simulink model "Simulatore_COMAU_UBHAND_IV_manipolazione_interna"  (Simulink 7.6)
settings: Solver=ode45  StartTime=0.0  StopTime=inf  RelTol=1e-3
values from neighbouring files (symbols this model uses):
  a_f = zeros(4,5)    [KinematicData.m]
  alpha_f = zeros(4,5)    [KinematicData.m]
  d_f = zeros(4,5)    [KinematicData.m]
  dc4_1 = 660    [KinematicData.m]
  T6HAND = [1 0 0 0;0 1 0 0; 0 0 1 357.48+95; 0 0 0 1]    [KinematicData.m]
  teta_d = atan(647.07/130)    [KinematicData.m]
  teta_f = zeros(4,5)    [KinematicData.m]
undefined symbols (not defined in the file or in its folder): a_c, a_w, alpha_c, alpha_w, d_c, d_w, Dt_fingers, teta_c
libraries: simulink x281, dspmtrx3 x171, aerolibutil x163, aerolibconvert2 x64, simulink_extras x46, vrlib x26, aerolibtransform2 x7, dspnetwork x1
(part 11 of 15)

== system Simulatore_COMAU_UBHAND_IV_manipolazione_interna/Work Space Control/Inverse_kinematic/Inverse_kinematic_comau/Subsystem/q5 appartiene a [-180 0]/q6 ==
  R_36 : Inport
  Gain1 : Gain  Gain=-1
  P06(1) : dspmtrx3/Submatrix  RowSpan=One row  RowStartMode=Index  RowStartIndex=3  RowEndMode=Index  RowEndIndex=3  ColSpan=One column  ColStartMode=Index  ColStartIndex=2  ColEndMode=Offset from last  ColEndIndex=1
  P06(1)1 : dspmtrx3/Submatrix  RowSpan=One row  RowStartMode=Index  RowStartIndex=3  RowEndMode=Index  RowEndIndex=3  ColSpan=One column  ColStartMode=Index  ColStartIndex=1  ColEndMode=Offset from last  ColEndIndex=1
  Trigonometric Function : Trigonometry  Operator=atan2
  q_6 : Outport
  wires:
  Trigonometric Function/1 -> q_6/1
  P06(1)/1 -> Gain1/1
  P06(1)1/1 -> Trigonometric Function/2
  R_36/1 -> P06(1)1/1, P06(1)/1
  Gain1/1 -> Trigonometric Function/1

== system Simulatore_COMAU_UBHAND_IV_manipolazione_interna/Work Space Control/Inverse_kinematic/Inverse_kinematic_comau/Subsystem/q5 appartiene a [0 180]/q4 ==
  R_36 : Inport
  P06(1) : dspmtrx3/Submatrix  RowSpan=One row  RowStartMode=Index  RowStartIndex=2  RowEndMode=Index  RowEndIndex=3  ColSpan=One column  ColStartMode=Index  ColStartIndex=3  ColEndMode=Offset from last  ColEndIndex=1
  P06(1)1 : dspmtrx3/Submatrix  RowSpan=One row  RowStartMode=Index  RowStartIndex=1  RowEndMode=Index  RowEndIndex=3  ColSpan=One column  ColStartMode=Index  ColStartIndex=3  ColEndMode=Offset from last  ColEndIndex=1
  Trigonometric Function : Trigonometry  Operator=atan2
  q_4 : Outport
  wires:
  R_36/1 -> P06(1)/1, P06(1)1/1
  P06(1)/1 -> Trigonometric Function/1
  P06(1)1/1 -> Trigonometric Function/2
  Trigonometric Function/1 -> q_4/1

== system Simulatore_COMAU_UBHAND_IV_manipolazione_interna/Work Space Control/Inverse_kinematic/Inverse_kinematic_comau/Subsystem/q5 appartiene a [0 180]/q5 ==
  R_36 : Inport
  Add : Sum
  Math Function : Math  Operator=sqrt  SaturateOnIntegerOverflow=on
  Math Function1 : Math  Operator=square  SaturateOnIntegerOverflow=on
  Math Function2 : Math  Operator=square  SaturateOnIntegerOverflow=on
  P06(1) : dspmtrx3/Submatrix  RowSpan=One row  RowStartMode=Index  RowStartIndex=1  RowEndMode=Index  RowEndIndex=3  ColSpan=One column  ColStartMode=Index  ColStartIndex=3  ColEndMode=Offset from last  ColEndIndex=1
  P06(1)1 : dspmtrx3/Submatrix  RowSpan=One row  RowStartMode=Index  RowStartIndex=2  RowEndMode=Index  RowEndIndex=3  ColSpan=One column  ColStartMode=Index  ColStartIndex=3  ColEndMode=Offset from last  ColEndIndex=1
  P06(1)2 : dspmtrx3/Submatrix  RowSpan=One row  RowStartMode=Index  RowStartIndex=3  RowEndMode=Index  RowEndIndex=3  ColSpan=One column  ColStartMode=Index  ColStartIndex=3  ColEndMode=Offset from last  ColEndIndex=1
  Trigonometric Function : Trigonometry  Operator=atan2
  q_5 : Outport
  wires:
  P06(1)1/1 -> Math Function2/1
  P06(1)/1 -> Math Function1/1
  R_36/1 -> P06(1)/1, P06(1)1/1, P06(1)2/1
  Math Function1/1 -> Add/1
  Math Function2/1 -> Add/2
  Add/1 -> Math Function/1
  P06(1)2/1 -> Trigonometric Function/2
  Math Function/1 -> Trigonometric Function/1
  Trigonometric Function/1 -> q_5/1

== system Simulatore_COMAU_UBHAND_IV_manipolazione_interna/Work Space Control/Inverse_kinematic/Inverse_kinematic_comau/Subsystem/q5 appartiene a [0 180]/q6 ==
  R_36 : Inport
  Gain : Gain  Gain=-1
  P06(1) : dspmtrx3/Submatrix  RowSpan=One row  RowStartMode=Index  RowStartIndex=3  RowEndMode=Index  RowEndIndex=3  ColSpan=One column  ColStartMode=Index  ColStartIndex=2  ColEndMode=Offset from last  ColEndIndex=1
  P06(1)1 : dspmtrx3/Submatrix  RowSpan=One row  RowStartMode=Index  RowStartIndex=3  RowEndMode=Index  RowEndIndex=3  ColSpan=One column  ColStartMode=Index  ColStartIndex=1  ColEndMode=Offset from last  ColEndIndex=1
  Trigonometric Function : Trigonometry  Operator=atan2
  q_6 : Outport
  wires:
  R_36/1 -> P06(1)/1, P06(1)1/1
  P06(1)1/1 -> Gain/1
  Gain/1 -> Trigonometric Function/2
  P06(1)/1 -> Trigonometric Function/1
  Trigonometric Function/1 -> q_6/1

== system Simulatore_COMAU_UBHAND_IV_manipolazione_interna/Work Space Control/Inverse_kinematic/Inverse_kinematic_hand/Inverse_kinematic_little/Direct_kinematic_index/Subsystem ==
  q : Inport
  alpha : Inport  Port=2
  a : Inport  Port=3
  A_01 : aerolibutil/Create 3x3 Matrix
  Constant : Constant  Value=0
  Constant1 : Constant  Value=[0 0 0 1]  VectorParams1D=off
  Constant5 : Constant  Value=0
  Display : Display
  Gain : Gain  Gain=-1
  Gain1 : Gain  Gain=-1
  Matrix Concatenation : Concatenate  Mode=Multidimensional array  ConcatenateDimension=2
  Matrix Concatenation1 : Concatenate  Mode=Multidimensional array
  Product{,1..5} (6 alike) : Product  RndMeth=Zero
  Trigonometric Function{,1,6..8,11..12} (7 alike) : Trigonometry  Operator=cos
  Trigonometric Function{2..5,9..10,13} (7 alike) : Trigonometry
  Vector Concatenate : Concatenate  NumInputs=3  Mode=Multidimensional array
  Out1 : Outport
  wires:
  Matrix Concatenation1/1 -> Display/1, Out1/1
  Constant1/1 -> Matrix Concatenation1/2
  Matrix Concatenation/1 -> Matrix Concatenation1/1
  Vector Concatenate/1 -> Matrix Concatenation/2
  A_01/1 -> Matrix Concatenation/1
  Constant5/1 -> Vector Concatenate/3
  Product5/1 -> Vector Concatenate/2
  Product4/1 -> Vector Concatenate/1
  Trigonometric Function13/1 -> Product5/2
  Trigonometric Function12/1 -> Product4/2
  a/1 -> Product5/1, Product4/1
  Trigonometric Function11/1 -> A_01/9
  Trigonometric Function10/1 -> A_01/8
  Constant/1 -> A_01/7
  Gain1/1 -> A_01/6
  Product2/1 -> A_01/5
  Trigonometric Function5/1 -> A_01/4
  Product1/1 -> A_01/3
  Gain/1 -> A_01/2
  Trigonometric Function/1 -> A_01/1
  Product/1 -> Gain/1
  Product3/1 -> Gain1/1
  Trigonometric Function9/1 -> Product3/2
  Trigonometric Function8/1 -> Product3/1
  Trigonometric Function7/1 -> Product2/2
  Trigonometric Function6/1 -> Product2/1
  Trigonometric Function4/1 -> Product1/2
  Trigonometric Function3/1 -> Product1/1
  alpha/1 -> Trigonometric Function11/1, Trigonometric Function10/1, Trigonometric Function9/1, Trigonometric Function7/1, Trigonometric Function4/1, Trigonometric Function1/1
  q/1 -> Trigonometric Function13/1, Trigonometric Function12/1, Trigonometric Function8/1, Trigonometric Function6/1, Trigonometric Function5/1, Trigonometric Function3/1, Trigonometric Function2/1, Trigonometric Function/1
  Trigonometric Function1/1 -> Product/2
  Trigonometric Function2/1 -> Product/1

== system Simulatore_COMAU_UBHAND_IV_manipolazione_interna/Work Space Control/Inverse_kinematic/Inverse_kinematic_hand/Inverse_kinematic_little/Direct_kinematic_index/Subsystem1 ==
  q : Inport
  alpha : Inport  Port=2
  a : Inport  Port=3
  A_01 : aerolibutil/Create 3x3 Matrix
  Constant : Constant  Value=0
  Constant1 : Constant  Value=[0 0 0 1]  VectorParams1D=off
  Constant5 : Constant  Value=0
  Display : Display
  Gain : Gain  Gain=-1
  Gain1 : Gain  Gain=-1
  Matrix Concatenation : Concatenate  Mode=Multidimensional array  ConcatenateDimension=2
  Matrix Concatenation1 : Concatenate  Mode=Multidimensional array
  Product{,1..5} (6 alike) : Product  RndMeth=Zero
  Trigonometric Function{,1,6..8,11..12} (7 alike) : Trigonometry  Operator=cos
  Trigonometric Function{2..5,9..10,13} (7 alike) : Trigonometry
  Vector Concatenate : Concatenate  NumInputs=3  Mode=Multidimensional array
  Out1 : Outport
  wires:
  Trigonometric Function2/1 -> Product/1
  Trigonometric Function1/1 -> Product/2
  q/1 -> Trigonometric Function/1, Trigonometric Function2/1, Trigonometric Function3/1, Trigonometric Function5/1, Trigonometric Function6/1, Trigonometric Function8/1, Trigonometric Function12/1, Trigonometric Function13/1
  alpha/1 -> Trigonometric Function1/1, Trigonometric Function4/1, Trigonometric Function7/1, Trigonometric Function9/1, Trigonometric Function10/1, Trigonometric Function11/1
  Trigonometric Function3/1 -> Product1/1
  Trigonometric Function4/1 -> Product1/2
  Trigonometric Function6/1 -> Product2/1
  Trigonometric Function7/1 -> Product2/2
  Trigonometric Function8/1 -> Product3/1
  Trigonometric Function9/1 -> Product3/2
  Product3/1 -> Gain1/1
  Product/1 -> Gain/1
  Trigonometric Function/1 -> A_01/1
  Gain/1 -> A_01/2
  Product1/1 -> A_01/3
  Trigonometric Function5/1 -> A_01/4
  Product2/1 -> A_01/5
  Gain1/1 -> A_01/6
  Constant/1 -> A_01/7
  Trigonometric Function10/1 -> A_01/8
  Trigonometric Function11/1 -> A_01/9
  a/1 -> Product5/1, Product4/1
  Trigonometric Function12/1 -> Product4/2
  Trigonometric Function13/1 -> Product5/2
  Product4/1 -> Vector Concatenate/1
  Product5/1 -> Vector Concatenate/2
  Constant5/1 -> Vector Concatenate/3
  A_01/1 -> Matrix Concatenation/1
  Vector Concatenate/1 -> Matrix Concatenation/2
  Matrix Concatenation/1 -> Matrix Concatenation1/1
  Constant1/1 -> Matrix Concatenation1/2
  Matrix Concatenation1/1 -> Out1/1, Display/1

== system Simulatore_COMAU_UBHAND_IV_manipolazione_interna/Work Space Control/Inverse_kinematic/Inverse_kinematic_hand/Inverse_kinematic_little/Direct_kinematic_index/Subsystem2 ==
  q : Inport
  alpha : Inport  Port=2
  a : Inport  Port=3
  A_01 : aerolibutil/Create 3x3 Matrix
  Constant : Constant  Value=0
  Constant1 : Constant  Value=[0 0 0 1]  VectorParams1D=off
  Constant5 : Constant  Value=0
  Display : Display
  Gain : Gain  Gain=-1
  Gain1 : Gain  Gain=-1
  Matrix Concatenation : Concatenate  Mode=Multidimensional array  ConcatenateDimension=2
  Matrix Concatenation1 : Concatenate  Mode=Multidimensional array
  Product{,1..5} (6 alike) : Product  RndMeth=Zero
  Trigonometric Function{,1,6..8,11..12} (7 alike) : Trigonometry  Operator=cos
  Trigonometric Function{2..5,9..10,13} (7 alike) : Trigonometry
  Vector Concatenate : Concatenate  NumInputs=3  Mode=Multidimensional array
  Out1 : Outport
  wires:
  Matrix Concatenation1/1 -> Display/1, Out1/1
  Constant1/1 -> Matrix Concatenation1/2
  Matrix Concatenation/1 -> Matrix Concatenation1/1
  Vector Concatenate/1 -> Matrix Concatenation/2
  A_01/1 -> Matrix Concatenation/1
  Constant5/1 -> Vector Concatenate/3
  Product5/1 -> Vector Concatenate/2
  Product4/1 -> Vector Concatenate/1
  Trigonometric Function13/1 -> Product5/2
  Trigonometric Function12/1 -> Product4/2
  a/1 -> Product5/1, Product4/1
  Trigonometric Function11/1 -> A_01/9
  Trigonometric Function10/1 -> A_01/8
  Constant/1 -> A_01/7
  Gain1/1 -> A_01/6
  Product2/1 -> A_01/5
  Trigonometric Function5/1 -> A_01/4
  Product1/1 -> A_01/3
  Gain/1 -> A_01/2
  Trigonometric Function/1 -> A_01/1
  Product/1 -> Gain/1
  Product3/1 -> Gain1/1
  Trigonometric Function9/1 -> Product3/2
  Trigonometric Function8/1 -> Product3/1
  Trigonometric Function7/1 -> Product2/2
  Trigonometric Function6/1 -> Product2/1
  Trigonometric Function4/1 -> Product1/2
  Trigonometric Function3/1 -> Product1/1
  alpha/1 -> Trigonometric Function11/1, Trigonometric Function10/1, Trigonometric Function9/1, Trigonometric Function7/1, Trigonometric Function4/1, Trigonometric Function1/1
  q/1 -> Trigonometric Function13/1, Trigonometric Function12/1, Trigonometric Function8/1, Trigonometric Function6/1, Trigonometric Function5/1, Trigonometric Function3/1, Trigonometric Function2/1, Trigonometric Function/1
  Trigonometric Function1/1 -> Product/2
  Trigonometric Function2/1 -> Product/1

== system Simulatore_COMAU_UBHAND_IV_manipolazione_interna/Work Space Control/Inverse_kinematic/Inverse_kinematic_hand/Inverse_kinematic_little/Direct_kinematic_index/Subsystem3 ==
  q : Inport
  alpha : Inport  Port=2
  a : Inport  Port=3
  A_01 : aerolibutil/Create 3x3 Matrix
  Constant : Constant  Value=0
  Constant1 : Constant  Value=[0 0 0 1]  VectorParams1D=off
  Constant5 : Constant  Value=0
  Display : Display
  Gain : Gain  Gain=-1
  Gain1 : Gain  Gain=-1
  Matrix Concatenation : Concatenate  Mode=Multidimensional array  ConcatenateDimension=2
  Matrix Concatenation1 : Concatenate  Mode=Multidimensional array
  Product{,1..5} (6 alike) : Product  RndMeth=Zero
  Trigonometric Function{,1,6..8,11..12} (7 alike) : Trigonometry  Operator=cos
  Trigonometric Function{2..5,9..10,13} (7 alike) : Trigonometry
  Vector Concatenate : Concatenate  NumInputs=3  Mode=Multidimensional array
  Out1 : Outport
  wires:
  Trigonometric Function2/1 -> Product/1
  Trigonometric Function1/1 -> Product/2
  q/1 -> Trigonometric Function/1, Trigonometric Function2/1, Trigonometric Function3/1, Trigonometric Function5/1, Trigonometric Function6/1, Trigonometric Function8/1, Trigonometric Function12/1, Trigonometric Function13/1
  alpha/1 -> Trigonometric Function1/1, Trigonometric Function4/1, Trigonometric Function7/1, Trigonometric Function9/1, Trigonometric Function10/1, Trigonometric Function11/1
  Trigonometric Function3/1 -> Product1/1
  Trigonometric Function4/1 -> Product1/2
  Trigonometric Function6/1 -> Product2/1
  Trigonometric Function7/1 -> Product2/2
  Trigonometric Function8/1 -> Product3/1
  Trigonometric Function9/1 -> Product3/2
  Product3/1 -> Gain1/1
  Product/1 -> Gain/1
  Trigonometric Function/1 -> A_01/1
  Gain/1 -> A_01/2
  Product1/1 -> A_01/3
  Trigonometric Function5/1 -> A_01/4
  Product2/1 -> A_01/5
  Gain1/1 -> A_01/6
  Constant/1 -> A_01/7
  Trigonometric Function10/1 -> A_01/8
  Trigonometric Function11/1 -> A_01/9
  a/1 -> Product5/1, Product4/1
  Trigonometric Function12/1 -> Product4/2
  Trigonometric Function13/1 -> Product5/2
  Product4/1 -> Vector Concatenate/1
  Product5/1 -> Vector Concatenate/2
  Constant5/1 -> Vector Concatenate/3
  A_01/1 -> Matrix Concatenation/1
  Vector Concatenate/1 -> Matrix Concatenation/2
  Matrix Concatenation/1 -> Matrix Concatenation1/1
  Constant1/1 -> Matrix Concatenation1/2
  Matrix Concatenation1/1 -> Out1/1, Display/1

== system Simulatore_COMAU_UBHAND_IV_manipolazione_interna/Work Space Control/Inverse_kinematic/Inverse_kinematic_hand/Inverse_kinematic_little/jacobian/z1 ==
  T_01 : Inport
  T_02 : Inport  Port=2
  T_03 : Inport  Port=3
  T_base : Inport  Port=4
  Cross Product : vrlib/Utilities/Cross Product
  Cross Product1 : vrlib/Utilities/Cross Product
  Cross Product2 : vrlib/Utilities/Cross Product
  pe-P(i-i) : Subsystem
  z(i-1) : Subsystem
  z0 : Outport
  z1 : Outport  Port=2
  z2 : Outport  Port=3
  z0xpe-p0 : Outport  Port=4
  z1xpe-p1 : Outport  Port=5
  z2xpe-p2 : Outport  Port=6
  wires:
  pe-P(i-i)/3(pe-p2) -> Cross Product2/2
  pe-P(i-i)/2(pe-p1) -> Cross Product1/2
  pe-P(i-i)/1(pe-p0) -> Cross Product/2
  z(i-1)/3(z2) -> Cross Product2/1, z2/1
  z(i-1)/2(z1) -> Cross Product1/1, z1/1
  z(i-1)/1(z0) -> Cross Product/1, z0/1
  Cross Product2/1 -> z2xpe-p2/1
  Cross Product1/1 -> z1xpe-p1/1
  Cross Product/1 -> z0xpe-p0/1
  T_base/1 -> pe-P(i-i)/4(T_base), z(i-1)/3(T_base)
  T_03/1 -> pe-P(i-i)/1(T_03)
  T_01/1 -> pe-P(i-i)/2(T_01), z(i-1)/1(T_01)
  T_02/1 -> pe-P(i-i)/3(T_02), z(i-1)/2(T_02)

== system Simulatore_COMAU_UBHAND_IV_manipolazione_interna/Work Space Control/Inverse_kinematic/Inverse_kinematic_hand/Inverse_kinematic_middle/Direct_kinematic_index/Subsystem ==
  q : Inport
  alpha : Inport  Port=2
  a : Inport  Port=3
  A_01 : aerolibutil/Create 3x3 Matrix
  Constant : Constant  Value=0
  Constant1 : Constant  Value=[0 0 0 1]  VectorParams1D=off
  Constant5 : Constant  Value=0
  Display : Display
  Gain : Gain  Gain=-1
  Gain1 : Gain  Gain=-1
  Matrix Concatenation : Concatenate  Mode=Multidimensional array  ConcatenateDimension=2
  Matrix Concatenation1 : Concatenate  Mode=Multidimensional array
  Product{,1..5} (6 alike) : Product  RndMeth=Zero
  Trigonometric Function{,1,6..8,11..12} (7 alike) : Trigonometry  Operator=cos
  Trigonometric Function{2..5,9..10,13} (7 alike) : Trigonometry
  Vector Concatenate : Concatenate  NumInputs=3  Mode=Multidimensional array
  Out1 : Outport
  wires:
  Matrix Concatenation1/1 -> Display/1, Out1/1
  Constant1/1 -> Matrix Concatenation1/2
  Matrix Concatenation/1 -> Matrix Concatenation1/1
  Vector Concatenate/1 -> Matrix Concatenation/2
  A_01/1 -> Matrix Concatenation/1
  Constant5/1 -> Vector Concatenate/3
  Product5/1 -> Vector Concatenate/2
  Product4/1 -> Vector Concatenate/1
  Trigonometric Function13/1 -> Product5/2
  Trigonometric Function12/1 -> Product4/2
  a/1 -> Product5/1, Product4/1
  Trigonometric Function11/1 -> A_01/9
  Trigonometric Function10/1 -> A_01/8
  Constant/1 -> A_01/7
  Gain1/1 -> A_01/6
  Product2/1 -> A_01/5
  Trigonometric Function5/1 -> A_01/4
  Product1/1 -> A_01/3
  Gain/1 -> A_01/2
  Trigonometric Function/1 -> A_01/1
  Product/1 -> Gain/1
  Product3/1 -> Gain1/1
  Trigonometric Function9/1 -> Product3/2
  Trigonometric Function8/1 -> Product3/1
  Trigonometric Function7/1 -> Product2/2
  Trigonometric Function6/1 -> Product2/1
  Trigonometric Function4/1 -> Product1/2
  Trigonometric Function3/1 -> Product1/1
  alpha/1 -> Trigonometric Function11/1, Trigonometric Function10/1, Trigonometric Function9/1, Trigonometric Function7/1, Trigonometric Function4/1, Trigonometric Function1/1
  q/1 -> Trigonometric Function13/1, Trigonometric Function12/1, Trigonometric Function8/1, Trigonometric Function6/1, Trigonometric Function5/1, Trigonometric Function3/1, Trigonometric Function2/1, Trigonometric Function/1
  Trigonometric Function1/1 -> Product/2
  Trigonometric Function2/1 -> Product/1

== system Simulatore_COMAU_UBHAND_IV_manipolazione_interna/Work Space Control/Inverse_kinematic/Inverse_kinematic_hand/Inverse_kinematic_middle/Direct_kinematic_index/Subsystem1 ==
  q : Inport
  alpha : Inport  Port=2
  a : Inport  Port=3
  A_01 : aerolibutil/Create 3x3 Matrix
  Constant : Constant  Value=0
  Constant1 : Constant  Value=[0 0 0 1]  VectorParams1D=off
  Constant5 : Constant  Value=0
  Display : Display
  Gain : Gain  Gain=-1
  Gain1 : Gain  Gain=-1
  Matrix Concatenation : Concatenate  Mode=Multidimensional array  ConcatenateDimension=2
  Matrix Concatenation1 : Concatenate  Mode=Multidimensional array
  Product{,1..5} (6 alike) : Product  RndMeth=Zero
  Trigonometric Function{,1,6..8,11..12} (7 alike) : Trigonometry  Operator=cos
  Trigonometric Function{2..5,9..10,13} (7 alike) : Trigonometry
  Vector Concatenate : Concatenate  NumInputs=3  Mode=Multidimensional array
  Out1 : Outport
  wires:
  Trigonometric Function2/1 -> Product/1
  Trigonometric Function1/1 -> Product/2
  q/1 -> Trigonometric Function/1, Trigonometric Function2/1, Trigonometric Function3/1, Trigonometric Function5/1, Trigonometric Function6/1, Trigonometric Function8/1, Trigonometric Function12/1, Trigonometric Function13/1
  alpha/1 -> Trigonometric Function1/1, Trigonometric Function4/1, Trigonometric Function7/1, Trigonometric Function9/1, Trigonometric Function10/1, Trigonometric Function11/1
  Trigonometric Function3/1 -> Product1/1
  Trigonometric Function4/1 -> Product1/2
  Trigonometric Function6/1 -> Product2/1
  Trigonometric Function7/1 -> Product2/2
  Trigonometric Function8/1 -> Product3/1
  Trigonometric Function9/1 -> Product3/2
  Product3/1 -> Gain1/1
  Product/1 -> Gain/1
  Trigonometric Function/1 -> A_01/1
  Gain/1 -> A_01/2
  Product1/1 -> A_01/3
  Trigonometric Function5/1 -> A_01/4
  Product2/1 -> A_01/5
  Gain1/1 -> A_01/6
  Constant/1 -> A_01/7
  Trigonometric Function10/1 -> A_01/8
  Trigonometric Function11/1 -> A_01/9
  a/1 -> Product5/1, Product4/1
  Trigonometric Function12/1 -> Product4/2
  Trigonometric Function13/1 -> Product5/2
  Product4/1 -> Vector Concatenate/1
  Product5/1 -> Vector Concatenate/2
  Constant5/1 -> Vector Concatenate/3
  A_01/1 -> Matrix Concatenation/1
  Vector Concatenate/1 -> Matrix Concatenation/2
  Matrix Concatenation/1 -> Matrix Concatenation1/1
  Constant1/1 -> Matrix Concatenation1/2
  Matrix Concatenation1/1 -> Out1/1, Display/1

== system Simulatore_COMAU_UBHAND_IV_manipolazione_interna/Work Space Control/Inverse_kinematic/Inverse_kinematic_hand/Inverse_kinematic_middle/Direct_kinematic_index/Subsystem2 ==
  q : Inport
  alpha : Inport  Port=2
  a : Inport  Port=3
  A_01 : aerolibutil/Create 3x3 Matrix
  Constant : Constant  Value=0
  Constant1 : Constant  Value=[0 0 0 1]  VectorParams1D=off
  Constant5 : Constant  Value=0
  Display : Display
  Gain : Gain  Gain=-1
  Gain1 : Gain  Gain=-1
  Matrix Concatenation : Concatenate  Mode=Multidimensional array  ConcatenateDimension=2
  Matrix Concatenation1 : Concatenate  Mode=Multidimensional array
  Product{,1..5} (6 alike) : Product  RndMeth=Zero
  Trigonometric Function{,1,6..8,11..12} (7 alike) : Trigonometry  Operator=cos
  Trigonometric Function{2..5,9..10,13} (7 alike) : Trigonometry
  Vector Concatenate : Concatenate  NumInputs=3  Mode=Multidimensional array
  Out1 : Outport
  wires:
  Matrix Concatenation1/1 -> Display/1, Out1/1
  Constant1/1 -> Matrix Concatenation1/2
  Matrix Concatenation/1 -> Matrix Concatenation1/1
  Vector Concatenate/1 -> Matrix Concatenation/2
  A_01/1 -> Matrix Concatenation/1
  Constant5/1 -> Vector Concatenate/3
  Product5/1 -> Vector Concatenate/2
  Product4/1 -> Vector Concatenate/1
  Trigonometric Function13/1 -> Product5/2
  Trigonometric Function12/1 -> Product4/2
  a/1 -> Product5/1, Product4/1
  Trigonometric Function11/1 -> A_01/9
  Trigonometric Function10/1 -> A_01/8
  Constant/1 -> A_01/7
  Gain1/1 -> A_01/6
  Product2/1 -> A_01/5
  Trigonometric Function5/1 -> A_01/4
  Product1/1 -> A_01/3
  Gain/1 -> A_01/2
  Trigonometric Function/1 -> A_01/1
  Product/1 -> Gain/1
  Product3/1 -> Gain1/1
  Trigonometric Function9/1 -> Product3/2
  Trigonometric Function8/1 -> Product3/1
  Trigonometric Function7/1 -> Product2/2
  Trigonometric Function6/1 -> Product2/1
  Trigonometric Function4/1 -> Product1/2
  Trigonometric Function3/1 -> Product1/1
  alpha/1 -> Trigonometric Function11/1, Trigonometric Function10/1, Trigonometric Function9/1, Trigonometric Function7/1, Trigonometric Function4/1, Trigonometric Function1/1
  q/1 -> Trigonometric Function13/1, Trigonometric Function12/1, Trigonometric Function8/1, Trigonometric Function6/1, Trigonometric Function5/1, Trigonometric Function3/1, Trigonometric Function2/1, Trigonometric Function/1
  Trigonometric Function1/1 -> Product/2
  Trigonometric Function2/1 -> Product/1

== system Simulatore_COMAU_UBHAND_IV_manipolazione_interna/Work Space Control/Inverse_kinematic/Inverse_kinematic_hand/Inverse_kinematic_middle/Direct_kinematic_index/Subsystem3 ==
  q : Inport
  alpha : Inport  Port=2
  a : Inport  Port=3
  A_01 : aerolibutil/Create 3x3 Matrix
  Constant : Constant  Value=0
  Constant1 : Constant  Value=[0 0 0 1]  VectorParams1D=off
  Constant5 : Constant  Value=0
  Display : Display
  Gain : Gain  Gain=-1
  Gain1 : Gain  Gain=-1
  Matrix Concatenation : Concatenate  Mode=Multidimensional array  ConcatenateDimension=2
  Matrix Concatenation1 : Concatenate  Mode=Multidimensional array
  Product{,1..5} (6 alike) : Product  RndMeth=Zero
  Trigonometric Function{,1,6..8,11..12} (7 alike) : Trigonometry  Operator=cos
  Trigonometric Function{2..5,9..10,13} (7 alike) : Trigonometry
  Vector Concatenate : Concatenate  NumInputs=3  Mode=Multidimensional array
  Out1 : Outport
  wires:
  Trigonometric Function2/1 -> Product/1
  Trigonometric Function1/1 -> Product/2
  q/1 -> Trigonometric Function/1, Trigonometric Function2/1, Trigonometric Function3/1, Trigonometric Function5/1, Trigonometric Function6/1, Trigonometric Function8/1, Trigonometric Function12/1, Trigonometric Function13/1
  alpha/1 -> Trigonometric Function1/1, Trigonometric Function4/1, Trigonometric Function7/1, Trigonometric Function9/1, Trigonometric Function10/1, Trigonometric Function11/1
  Trigonometric Function3/1 -> Product1/1
  Trigonometric Function4/1 -> Product1/2
  Trigonometric Function6/1 -> Product2/1
  Trigonometric Function7/1 -> Product2/2
  Trigonometric Function8/1 -> Product3/1
  Trigonometric Function9/1 -> Product3/2
  Product3/1 -> Gain1/1
  Product/1 -> Gain/1
  Trigonometric Function/1 -> A_01/1
  Gain/1 -> A_01/2
  Product1/1 -> A_01/3
  Trigonometric Function5/1 -> A_01/4
  Product2/1 -> A_01/5
  Gain1/1 -> A_01/6
  Constant/1 -> A_01/7
  Trigonometric Function10/1 -> A_01/8
  Trigonometric Function11/1 -> A_01/9
  a/1 -> Product5/1, Product4/1
  Trigonometric Function12/1 -> Product4/2
  Trigonometric Function13/1 -> Product5/2
  Product4/1 -> Vector Concatenate/1
  Product5/1 -> Vector Concatenate/2
  Constant5/1 -> Vector Concatenate/3
  A_01/1 -> Matrix Concatenation/1
  Vector Concatenate/1 -> Matrix Concatenation/2
  Matrix Concatenation/1 -> Matrix Concatenation1/1
  Constant1/1 -> Matrix Concatenation1/2
  Matrix Concatenation1/1 -> Out1/1, Display/1

== system Simulatore_COMAU_UBHAND_IV_manipolazione_interna/Work Space Control/Inverse_kinematic/Inverse_kinematic_hand/Inverse_kinematic_middle/jacobian/z1 ==
  T_01 : Inport
  T_02 : Inport  Port=2
  T_03 : Inport  Port=3
  T_base : Inport  Port=4
  Cross Product : vrlib/Utilities/Cross Product
  Cross Product1 : vrlib/Utilities/Cross Product
  Cross Product2 : vrlib/Utilities/Cross Product
  pe-P(i-i) : Subsystem
  z(i-1) : Subsystem
  z0 : Outport
  z1 : Outport  Port=2
  z2 : Outport  Port=3
  z0xpe-p0 : Outport  Port=4
  z1xpe-p1 : Outport  Port=5
  z2xpe-p2 : Outport  Port=6
  wires:
  pe-P(i-i)/3(pe-p2) -> Cross Product2/2
  pe-P(i-i)/2(pe-p1) -> Cross Product1/2
  pe-P(i-i)/1(pe-p0) -> Cross Product/2
  z(i-1)/3(z2) -> Cross Product2/1, z2/1
  z(i-1)/2(z1) -> Cross Product1/1, z1/1
  z(i-1)/1(z0) -> Cross Product/1, z0/1
  Cross Product2/1 -> z2xpe-p2/1
  Cross Product1/1 -> z1xpe-p1/1
  Cross Product/1 -> z0xpe-p0/1
  T_base/1 -> pe-P(i-i)/4(T_base), z(i-1)/3(T_base)
  T_03/1 -> pe-P(i-i)/1(T_03)
  T_01/1 -> pe-P(i-i)/2(T_01), z(i-1)/1(T_01)
  T_02/1 -> pe-P(i-i)/3(T_02), z(i-1)/2(T_02)

== system Simulatore_COMAU_UBHAND_IV_manipolazione_interna/Work Space Control/Inverse_kinematic/Inverse_kinematic_hand/Inverse_kinematic_middle1/Direct_kinematic_index/Subsystem ==
  q : Inport
  alpha : Inport  Port=2
  a : Inport  Port=3
  A_01 : aerolibutil/Create 3x3 Matrix
  Constant : Constant  Value=0
  Constant1 : Constant  Value=[0 0 0 1]  VectorParams1D=off
  Constant5 : Constant  Value=0
  Display : Display
  Gain : Gain  Gain=-1
  Gain1 : Gain  Gain=-1
  Matrix Concatenation : Concatenate  Mode=Multidimensional array  ConcatenateDimension=2
  Matrix Concatenation1 : Concatenate  Mode=Multidimensional array
  Product{,1..5} (6 alike) : Product  RndMeth=Zero
  Trigonometric Function{,1,6..8,11..12} (7 alike) : Trigonometry  Operator=cos
  Trigonometric Function{2..5,9..10,13} (7 alike) : Trigonometry
  Vector Concatenate : Concatenate  NumInputs=3  Mode=Multidimensional array
  Out1 : Outport
  wires:
  Matrix Concatenation1/1 -> Display/1, Out1/1
  Constant1/1 -> Matrix Concatenation1/2
  Matrix Concatenation/1 -> Matrix Concatenation1/1
  Vector Concatenate/1 -> Matrix Concatenation/2
  A_01/1 -> Matrix Concatenation/1
  Constant5/1 -> Vector Concatenate/3
  Product5/1 -> Vector Concatenate/2
  Product4/1 -> Vector Concatenate/1
  Trigonometric Function13/1 -> Product5/2
  Trigonometric Function12/1 -> Product4/2
  a/1 -> Product5/1, Product4/1
  Trigonometric Function11/1 -> A_01/9
  Trigonometric Function10/1 -> A_01/8
  Constant/1 -> A_01/7
  Gain1/1 -> A_01/6
  Product2/1 -> A_01/5
  Trigonometric Function5/1 -> A_01/4
  Product1/1 -> A_01/3
  Gain/1 -> A_01/2
  Trigonometric Function/1 -> A_01/1
  Product/1 -> Gain/1
  Product3/1 -> Gain1/1
  Trigonometric Function9/1 -> Product3/2
  Trigonometric Function8/1 -> Product3/1
  Trigonometric Function7/1 -> Product2/2
  Trigonometric Function6/1 -> Product2/1
  Trigonometric Function4/1 -> Product1/2
  Trigonometric Function3/1 -> Product1/1
  alpha/1 -> Trigonometric Function11/1, Trigonometric Function10/1, Trigonometric Function9/1, Trigonometric Function7/1, Trigonometric Function4/1, Trigonometric Function1/1
  q/1 -> Trigonometric Function13/1, Trigonometric Function12/1, Trigonometric Function8/1, Trigonometric Function6/1, Trigonometric Function5/1, Trigonometric Function3/1, Trigonometric Function2/1, Trigonometric Function/1
  Trigonometric Function1/1 -> Product/2
  Trigonometric Function2/1 -> Product/1

== system Simulatore_COMAU_UBHAND_IV_manipolazione_interna/Work Space Control/Inverse_kinematic/Inverse_kinematic_hand/Inverse_kinematic_middle1/Direct_kinematic_index/Subsystem1 ==
  q : Inport
  alpha : Inport  Port=2
  a : Inport  Port=3
  A_01 : aerolibutil/Create 3x3 Matrix
  Constant : Constant  Value=0
  Constant1 : Constant  Value=[0 0 0 1]  VectorParams1D=off
  Constant5 : Constant  Value=0
  Display : Display
  Gain : Gain  Gain=-1
  Gain1 : Gain  Gain=-1
  Matrix Concatenation : Concatenate  Mode=Multidimensional array  ConcatenateDimension=2
  Matrix Concatenation1 : Concatenate  Mode=Multidimensional array
  Product{,1..5} (6 alike) : Product  RndMeth=Zero
  Trigonometric Function{,1,6..8,11..12} (7 alike) : Trigonometry  Operator=cos
  Trigonometric Function{2..5,9..10,13} (7 alike) : Trigonometry
  Vector Concatenate : Concatenate  NumInputs=3  Mode=Multidimensional array
  Out1 : Outport
  wires:
  Trigonometric Function2/1 -> Product/1
  Trigonometric Function1/1 -> Product/2
  q/1 -> Trigonometric Function/1, Trigonometric Function2/1, Trigonometric Function3/1, Trigonometric Function5/1, Trigonometric Function6/1, Trigonometric Function8/1, Trigonometric Function12/1, Trigonometric Function13/1
  alpha/1 -> Trigonometric Function1/1, Trigonometric Function4/1, Trigonometric Function7/1, Trigonometric Function9/1, Trigonometric Function10/1, Trigonometric Function11/1
  Trigonometric Function3/1 -> Product1/1
  Trigonometric Function4/1 -> Product1/2
  Trigonometric Function6/1 -> Product2/1
  Trigonometric Function7/1 -> Product2/2
  Trigonometric Function8/1 -> Product3/1
  Trigonometric Function9/1 -> Product3/2
  Product3/1 -> Gain1/1
  Product/1 -> Gain/1
  Trigonometric Function/1 -> A_01/1
  Gain/1 -> A_01/2
  Product1/1 -> A_01/3
  Trigonometric Function5/1 -> A_01/4
  Product2/1 -> A_01/5
  Gain1/1 -> A_01/6
  Constant/1 -> A_01/7
  Trigonometric Function10/1 -> A_01/8
  Trigonometric Function11/1 -> A_01/9
  a/1 -> Product5/1, Product4/1
  Trigonometric Function12/1 -> Product4/2
  Trigonometric Function13/1 -> Product5/2
  Product4/1 -> Vector Concatenate/1
  Product5/1 -> Vector Concatenate/2
  Constant5/1 -> Vector Concatenate/3
  A_01/1 -> Matrix Concatenation/1
  Vector Concatenate/1 -> Matrix Concatenation/2
  Matrix Concatenation/1 -> Matrix Concatenation1/1
  Constant1/1 -> Matrix Concatenation1/2
  Matrix Concatenation1/1 -> Out1/1, Display/1

== system Simulatore_COMAU_UBHAND_IV_manipolazione_interna/Work Space Control/Inverse_kinematic/Inverse_kinematic_hand/Inverse_kinematic_middle1/Direct_kinematic_index/Subsystem2 ==
  q : Inport
  alpha : Inport  Port=2
  a : Inport  Port=3
  A_01 : aerolibutil/Create 3x3 Matrix
  Constant : Constant  Value=0
  Constant1 : Constant  Value=[0 0 0 1]  VectorParams1D=off
  Constant5 : Constant  Value=0
  Display : Display
  Gain : Gain  Gain=-1
  Gain1 : Gain  Gain=-1
  Matrix Concatenation : Concatenate  Mode=Multidimensional array  ConcatenateDimension=2
  Matrix Concatenation1 : Concatenate  Mode=Multidimensional array
  Product{,1..5} (6 alike) : Product  RndMeth=Zero
  Trigonometric Function{,1,6..8,11..12} (7 alike) : Trigonometry  Operator=cos
  Trigonometric Function{2..5,9..10,13} (7 alike) : Trigonometry
  Vector Concatenate : Concatenate  NumInputs=3  Mode=Multidimensional array
  Out1 : Outport
  wires:
  Matrix Concatenation1/1 -> Display/1, Out1/1
  Constant1/1 -> Matrix Concatenation1/2
  Matrix Concatenation/1 -> Matrix Concatenation1/1
  Vector Concatenate/1 -> Matrix Concatenation/2
  A_01/1 -> Matrix Concatenation/1
  Constant5/1 -> Vector Concatenate/3
  Product5/1 -> Vector Concatenate/2
  Product4/1 -> Vector Concatenate/1
  Trigonometric Function13/1 -> Product5/2
  Trigonometric Function12/1 -> Product4/2
  a/1 -> Product5/1, Product4/1
  Trigonometric Function11/1 -> A_01/9
  Trigonometric Function10/1 -> A_01/8
  Constant/1 -> A_01/7
  Gain1/1 -> A_01/6
  Product2/1 -> A_01/5
  Trigonometric Function5/1 -> A_01/4
  Product1/1 -> A_01/3
  Gain/1 -> A_01/2
  Trigonometric Function/1 -> A_01/1
  Product/1 -> Gain/1
  Product3/1 -> Gain1/1
  Trigonometric Function9/1 -> Product3/2
  Trigonometric Function8/1 -> Product3/1
  Trigonometric Function7/1 -> Product2/2
  Trigonometric Function6/1 -> Product2/1
  Trigonometric Function4/1 -> Product1/2
  Trigonometric Function3/1 -> Product1/1
  alpha/1 -> Trigonometric Function11/1, Trigonometric Function10/1, Trigonometric Function9/1, Trigonometric Function7/1, Trigonometric Function4/1, Trigonometric Function1/1
  q/1 -> Trigonometric Function13/1, Trigonometric Function12/1, Trigonometric Function8/1, Trigonometric Function6/1, Trigonometric Function5/1, Trigonometric Function3/1, Trigonometric Function2/1, Trigonometric Function/1
  Trigonometric Function1/1 -> Product/2
  Trigonometric Function2/1 -> Product/1

== system Simulatore_COMAU_UBHAND_IV_manipolazione_interna/Work Space Control/Inverse_kinematic/Inverse_kinematic_hand/Inverse_kinematic_middle1/Direct_kinematic_index/Subsystem3 ==
  q : Inport
  alpha : Inport  Port=2
  a : Inport  Port=3
  A_01 : aerolibutil/Create 3x3 Matrix
  Constant : Constant  Value=0
  Constant1 : Constant  Value=[0 0 0 1]  VectorParams1D=off
  Constant5 : Constant  Value=0
  Display : Display
  Gain : Gain  Gain=-1
  Gain1 : Gain  Gain=-1
  Matrix Concatenation : Concatenate  Mode=Multidimensional array  ConcatenateDimension=2
  Matrix Concatenation1 : Concatenate  Mode=Multidimensional array
  Product{,1..5} (6 alike) : Product  RndMeth=Zero
  Trigonometric Function{,1,6..8,11..12} (7 alike) : Trigonometry  Operator=cos
  Trigonometric Function{2..5,9..10,13} (7 alike) : Trigonometry
  Vector Concatenate : Concatenate  NumInputs=3  Mode=Multidimensional array
  Out1 : Outport
  wires:
  Trigonometric Function2/1 -> Product/1
  Trigonometric Function1/1 -> Product/2
  q/1 -> Trigonometric Function/1, Trigonometric Function2/1, Trigonometric Function3/1, Trigonometric Function5/1, Trigonometric Function6/1, Trigonometric Function8/1, Trigonometric Function12/1, Trigonometric Function13/1
  alpha/1 -> Trigonometric Function1/1, Trigonometric Function4/1, Trigonometric Function7/1, Trigonometric Function9/1, Trigonometric Function10/1, Trigonometric Function11/1
  Trigonometric Function3/1 -> Product1/1
  Trigonometric Function4/1 -> Product1/2
  Trigonometric Function6/1 -> Product2/1
  Trigonometric Function7/1 -> Product2/2
  Trigonometric Function8/1 -> Product3/1
  Trigonometric Function9/1 -> Product3/2
  Product3/1 -> Gain1/1
  Product/1 -> Gain/1
  Trigonometric Function/1 -> A_01/1
  Gain/1 -> A_01/2
  Product1/1 -> A_01/3
  Trigonometric Function5/1 -> A_01/4
  Product2/1 -> A_01/5
  Gain1/1 -> A_01/6
  Constant/1 -> A_01/7
  Trigonometric Function10/1 -> A_01/8
  Trigonometric Function11/1 -> A_01/9
  a/1 -> Product5/1, Product4/1
  Trigonometric Function12/1 -> Product4/2
  Trigonometric Function13/1 -> Product5/2
  Product4/1 -> Vector Concatenate/1
  Product5/1 -> Vector Concatenate/2
  Constant5/1 -> Vector Concatenate/3
  A_01/1 -> Matrix Concatenation/1
  Vector Concatenate/1 -> Matrix Concatenation/2
  Matrix Concatenation/1 -> Matrix Concatenation1/1
  Constant1/1 -> Matrix Concatenation1/2
  Matrix Concatenation1/1 -> Out1/1, Display/1

== system Simulatore_COMAU_UBHAND_IV_manipolazione_interna/Work Space Control/Inverse_kinematic/Inverse_kinematic_hand/Inverse_kinematic_middle1/jacobian/z1 ==
  T_01 : Inport
  T_02 : Inport  Port=2
  T_03 : Inport  Port=3
  T_base : Inport  Port=4
  Cross Product : vrlib/Utilities/Cross Product
  Cross Product1 : vrlib/Utilities/Cross Product
  Cross Product2 : vrlib/Utilities/Cross Product
  pe-P(i-i) : Subsystem
  z(i-1) : Subsystem
  z0 : Outport
  z1 : Outport  Port=2
  z2 : Outport  Port=3
  z0xpe-p0 : Outport  Port=4
  z1xpe-p1 : Outport  Port=5
  z2xpe-p2 : Outport  Port=6
  wires:
  pe-P(i-i)/3(pe-p2) -> Cross Product2/2
  pe-P(i-i)/2(pe-p1) -> Cross Product1/2
  pe-P(i-i)/1(pe-p0) -> Cross Product/2
  z(i-1)/3(z2) -> Cross Product2/1, z2/1
  z(i-1)/2(z1) -> Cross Product1/1, z1/1
  z(i-1)/1(z0) -> Cross Product/1, z0/1
  Cross Product2/1 -> z2xpe-p2/1
  Cross Product1/1 -> z1xpe-p1/1
  Cross Product/1 -> z0xpe-p0/1
  T_base/1 -> pe-P(i-i)/4(T_base), z(i-1)/3(T_base)
  T_03/1 -> pe-P(i-i)/1(T_03)
  T_01/1 -> pe-P(i-i)/2(T_01), z(i-1)/1(T_01)
  T_02/1 -> pe-P(i-i)/3(T_02), z(i-1)/2(T_02)

== system Simulatore_COMAU_UBHAND_IV_manipolazione_interna/Work Space Control/Inverse_kinematic/Inverse_kinematic_hand/Inverse_kinematic_ring/Direct_kinematic_index/Subsystem ==
  q : Inport
  alpha : Inport  Port=2
  a : Inport  Port=3
  A_01 : aerolibutil/Create 3x3 Matrix
  Constant : Constant  Value=0
  Constant1 : Constant  Value=[0 0 0 1]  VectorParams1D=off
  Constant5 : Constant  Value=0
  Display : Display
  Gain : Gain  Gain=-1
  Gain1 : Gain  Gain=-1
  Matrix Concatenation : Concatenate  Mode=Multidimensional array  ConcatenateDimension=2
  Matrix Concatenation1 : Concatenate  Mode=Multidimensional array
  Product{,1..5} (6 alike) : Product  RndMeth=Zero
  Trigonometric Function{,1,6..8,11..12} (7 alike) : Trigonometry  Operator=cos
  Trigonometric Function{2..5,9..10,13} (7 alike) : Trigonometry
  Vector Concatenate : Concatenate  NumInputs=3  Mode=Multidimensional array
  Out1 : Outport
  wires:
  Matrix Concatenation1/1 -> Display/1, Out1/1
  Constant1/1 -> Matrix Concatenation1/2
  Matrix Concatenation/1 -> Matrix Concatenation1/1
  Vector Concatenate/1 -> Matrix Concatenation/2
  A_01/1 -> Matrix Concatenation/1
  Constant5/1 -> Vector Concatenate/3
  Product5/1 -> Vector Concatenate/2
  Product4/1 -> Vector Concatenate/1
  Trigonometric Function13/1 -> Product5/2
  Trigonometric Function12/1 -> Product4/2
  a/1 -> Product5/1, Product4/1
  Trigonometric Function11/1 -> A_01/9
  Trigonometric Function10/1 -> A_01/8
  Constant/1 -> A_01/7
  Gain1/1 -> A_01/6
  Product2/1 -> A_01/5
  Trigonometric Function5/1 -> A_01/4
  Product1/1 -> A_01/3
  Gain/1 -> A_01/2
  Trigonometric Function/1 -> A_01/1
  Product/1 -> Gain/1
  Product3/1 -> Gain1/1
  Trigonometric Function9/1 -> Product3/2
  Trigonometric Function8/1 -> Product3/1
  Trigonometric Function7/1 -> Product2/2
  Trigonometric Function6/1 -> Product2/1
  Trigonometric Function4/1 -> Product1/2
  Trigonometric Function3/1 -> Product1/1
  alpha/1 -> Trigonometric Function11/1, Trigonometric Function10/1, Trigonometric Function9/1, Trigonometric Function7/1, Trigonometric Function4/1, Trigonometric Function1/1
  q/1 -> Trigonometric Function13/1, Trigonometric Function12/1, Trigonometric Function8/1, Trigonometric Function6/1, Trigonometric Function5/1, Trigonometric Function3/1, Trigonometric Function2/1, Trigonometric Function/1
  Trigonometric Function1/1 -> Product/2
  Trigonometric Function2/1 -> Product/1

== system Simulatore_COMAU_UBHAND_IV_manipolazione_interna/Work Space Control/Inverse_kinematic/Inverse_kinematic_hand/Inverse_kinematic_ring/Direct_kinematic_index/Subsystem1 ==
  q : Inport
  alpha : Inport  Port=2
  a : Inport  Port=3
  A_01 : aerolibutil/Create 3x3 Matrix
  Constant : Constant  Value=0
  Constant1 : Constant  Value=[0 0 0 1]  VectorParams1D=off
  Constant5 : Constant  Value=0
  Display : Display
  Gain : Gain  Gain=-1
  Gain1 : Gain  Gain=-1
  Matrix Concatenation : Concatenate  Mode=Multidimensional array  ConcatenateDimension=2
  Matrix Concatenation1 : Concatenate  Mode=Multidimensional array
  Product{,1..5} (6 alike) : Product  RndMeth=Zero
  Trigonometric Function{,1,6..8,11..12} (7 alike) : Trigonometry  Operator=cos
  Trigonometric Function{2..5,9..10,13} (7 alike) : Trigonometry
  Vector Concatenate : Concatenate  NumInputs=3  Mode=Multidimensional array
  Out1 : Outport
  wires:
  Trigonometric Function2/1 -> Product/1
  Trigonometric Function1/1 -> Product/2
  q/1 -> Trigonometric Function/1, Trigonometric Function2/1, Trigonometric Function3/1, Trigonometric Function5/1, Trigonometric Function6/1, Trigonometric Function8/1, Trigonometric Function12/1, Trigonometric Function13/1
  alpha/1 -> Trigonometric Function1/1, Trigonometric Function4/1, Trigonometric Function7/1, Trigonometric Function9/1, Trigonometric Function10/1, Trigonometric Function11/1
  Trigonometric Function3/1 -> Product1/1
  Trigonometric Function4/1 -> Product1/2
  Trigonometric Function6/1 -> Product2/1
  Trigonometric Function7/1 -> Product2/2
  Trigonometric Function8/1 -> Product3/1
  Trigonometric Function9/1 -> Product3/2
  Product3/1 -> Gain1/1
  Product/1 -> Gain/1
  Trigonometric Function/1 -> A_01/1
  Gain/1 -> A_01/2
  Product1/1 -> A_01/3
  Trigonometric Function5/1 -> A_01/4
  Product2/1 -> A_01/5
  Gain1/1 -> A_01/6
  Constant/1 -> A_01/7
  Trigonometric Function10/1 -> A_01/8
  Trigonometric Function11/1 -> A_01/9
  a/1 -> Product5/1, Product4/1
  Trigonometric Function12/1 -> Product4/2
  Trigonometric Function13/1 -> Product5/2
  Product4/1 -> Vector Concatenate/1
  Product5/1 -> Vector Concatenate/2
  Constant5/1 -> Vector Concatenate/3
  A_01/1 -> Matrix Concatenation/1
  Vector Concatenate/1 -> Matrix Concatenation/2
  Matrix Concatenation/1 -> Matrix Concatenation1/1
  Constant1/1 -> Matrix Concatenation1/2
  Matrix Concatenation1/1 -> Out1/1, Display/1

== system Simulatore_COMAU_UBHAND_IV_manipolazione_interna/Work Space Control/Inverse_kinematic/Inverse_kinematic_hand/Inverse_kinematic_ring/Direct_kinematic_index/Subsystem2 ==
  q : Inport
  alpha : Inport  Port=2
  a : Inport  Port=3
  A_01 : aerolibutil/Create 3x3 Matrix
  Constant : Constant  Value=0
  Constant1 : Constant  Value=[0 0 0 1]  VectorParams1D=off
  Constant5 : Constant  Value=0
  Display : Display
  Gain : Gain  Gain=-1
  Gain1 : Gain  Gain=-1
  Matrix Concatenation : Concatenate  Mode=Multidimensional array  ConcatenateDimension=2
  Matrix Concatenation1 : Concatenate  Mode=Multidimensional array
  Product{,1..5} (6 alike) : Product  RndMeth=Zero
  Trigonometric Function{,1,6..8,11..12} (7 alike) : Trigonometry  Operator=cos
  Trigonometric Function{2..5,9..10,13} (7 alike) : Trigonometry
  Vector Concatenate : Concatenate  NumInputs=3  Mode=Multidimensional array
  Out1 : Outport
  wires:
  Matrix Concatenation1/1 -> Display/1, Out1/1
  Constant1/1 -> Matrix Concatenation1/2
  Matrix Concatenation/1 -> Matrix Concatenation1/1
  Vector Concatenate/1 -> Matrix Concatenation/2
  A_01/1 -> Matrix Concatenation/1
  Constant5/1 -> Vector Concatenate/3
  Product5/1 -> Vector Concatenate/2
  Product4/1 -> Vector Concatenate/1
  Trigonometric Function13/1 -> Product5/2
  Trigonometric Function12/1 -> Product4/2
  a/1 -> Product5/1, Product4/1
  Trigonometric Function11/1 -> A_01/9
  Trigonometric Function10/1 -> A_01/8
  Constant/1 -> A_01/7
  Gain1/1 -> A_01/6
  Product2/1 -> A_01/5
  Trigonometric Function5/1 -> A_01/4
  Product1/1 -> A_01/3
  Gain/1 -> A_01/2
  Trigonometric Function/1 -> A_01/1
  Product/1 -> Gain/1
  Product3/1 -> Gain1/1
  Trigonometric Function9/1 -> Product3/2
  Trigonometric Function8/1 -> Product3/1
  Trigonometric Function7/1 -> Product2/2
  Trigonometric Function6/1 -> Product2/1
  Trigonometric Function4/1 -> Product1/2
  Trigonometric Function3/1 -> Product1/1
  alpha/1 -> Trigonometric Function11/1, Trigonometric Function10/1, Trigonometric Function9/1, Trigonometric Function7/1, Trigonometric Function4/1, Trigonometric Function1/1
  q/1 -> Trigonometric Function13/1, Trigonometric Function12/1, Trigonometric Function8/1, Trigonometric Function6/1, Trigonometric Function5/1, Trigonometric Function3/1, Trigonometric Function2/1, Trigonometric Function/1
  Trigonometric Function1/1 -> Product/2
  Trigonometric Function2/1 -> Product/1

== system Simulatore_COMAU_UBHAND_IV_manipolazione_interna/Work Space Control/Inverse_kinematic/Inverse_kinematic_hand/Inverse_kinematic_ring/Direct_kinematic_index/Subsystem3 ==
  q : Inport
  alpha : Inport  Port=2
  a : Inport  Port=3
  A_01 : aerolibutil/Create 3x3 Matrix
  Constant : Constant  Value=0
  Constant1 : Constant  Value=[0 0 0 1]  VectorParams1D=off
  Constant5 : Constant  Value=0
  Display : Display
  Gain : Gain  Gain=-1
  Gain1 : Gain  Gain=-1
  Matrix Concatenation : Concatenate  Mode=Multidimensional array  ConcatenateDimension=2
  Matrix Concatenation1 : Concatenate  Mode=Multidimensional array
  Product{,1..5} (6 alike) : Product  RndMeth=Zero
  Trigonometric Function{,1,6..8,11..12} (7 alike) : Trigonometry  Operator=cos
  Trigonometric Function{2..5,9..10,13} (7 alike) : Trigonometry
  Vector Concatenate : Concatenate  NumInputs=3  Mode=Multidimensional array
  Out1 : Outport
  wires:
  Trigonometric Function2/1 -> Product/1
  Trigonometric Function1/1 -> Product/2
  q/1 -> Trigonometric Function/1, Trigonometric Function2/1, Trigonometric Function3/1, Trigonometric Function5/1, Trigonometric Function6/1, Trigonometric Function8/1, Trigonometric Function12/1, Trigonometric Function13/1
  alpha/1 -> Trigonometric Function1/1, Trigonometric Function4/1, Trigonometric Function7/1, Trigonometric Function9/1, Trigonometric Function10/1, Trigonometric Function11/1
  Trigonometric Function3/1 -> Product1/1
  Trigonometric Function4/1 -> Product1/2
  Trigonometric Function6/1 -> Product2/1
  Trigonometric Function7/1 -> Product2/2
  Trigonometric Function8/1 -> Product3/1
  Trigonometric Function9/1 -> Product3/2
  Product3/1 -> Gain1/1
  Product/1 -> Gain/1
  Trigonometric Function/1 -> A_01/1
  Gain/1 -> A_01/2
  Product1/1 -> A_01/3
  Trigonometric Function5/1 -> A_01/4
  Product2/1 -> A_01/5
  Gain1/1 -> A_01/6
  Constant/1 -> A_01/7
  Trigonometric Function10/1 -> A_01/8
  Trigonometric Function11/1 -> A_01/9
  a/1 -> Product5/1, Product4/1
  Trigonometric Function12/1 -> Product4/2
  Trigonometric Function13/1 -> Product5/2
  Product4/1 -> Vector Concatenate/1
  Product5/1 -> Vector Concatenate/2
  Constant5/1 -> Vector Concatenate/3
  A_01/1 -> Matrix Concatenation/1
  Vector Concatenate/1 -> Matrix Concatenation/2
  Matrix Concatenation/1 -> Matrix Concatenation1/1
  Constant1/1 -> Matrix Concatenation1/2
  Matrix Concatenation1/1 -> Out1/1, Display/1

== system Simulatore_COMAU_UBHAND_IV_manipolazione_interna/Work Space Control/Inverse_kinematic/Inverse_kinematic_hand/Inverse_kinematic_ring/jacobian/z1 ==
  T_01 : Inport
  T_02 : Inport  Port=2
  T_03 : Inport  Port=3
  T_base : Inport  Port=4
  Cross Product : vrlib/Utilities/Cross Product
  Cross Product1 : vrlib/Utilities/Cross Product
  Cross Product2 : vrlib/Utilities/Cross Product
  pe-P(i-i) : Subsystem
  z(i-1) : Subsystem
  z0 : Outport
  z1 : Outport  Port=2
  z2 : Outport  Port=3
  z0xpe-p0 : Outport  Port=4
  z1xpe-p1 : Outport  Port=5
  z2xpe-p2 : Outport  Port=6
  wires:
  pe-P(i-i)/3(pe-p2) -> Cross Product2/2
  pe-P(i-i)/2(pe-p1) -> Cross Product1/2
  pe-P(i-i)/1(pe-p0) -> Cross Product/2
  z(i-1)/3(z2) -> Cross Product2/1, z2/1
  z(i-1)/2(z1) -> Cross Product1/1, z1/1
  z(i-1)/1(z0) -> Cross Product/1, z0/1
  Cross Product2/1 -> z2xpe-p2/1
  Cross Product1/1 -> z1xpe-p1/1
  Cross Product/1 -> z0xpe-p0/1
  T_base/1 -> pe-P(i-i)/4(T_base), z(i-1)/3(T_base)
  T_03/1 -> pe-P(i-i)/1(T_03)
  T_01/1 -> pe-P(i-i)/2(T_01), z(i-1)/1(T_01)
  T_02/1 -> pe-P(i-i)/3(T_02), z(i-1)/2(T_02)
